simulink model "V2x_TC_TrafficLights_cs"  (Simulink 7.6)
settings: Solver=ode5  SolverMode=SingleTasking  StartTime=0.0  StopTime=38  FixedStep=1/100  RelTol=1e-3
PreLoadFcn:
  | check_NUTS_defined
  | 
  | assignin('base',['model_closed_' bdroot],false);addpath('../includes_V2x');
InitFcn:
  | PreScanExperimentInitFcn(bdroot)
StopFcn:
  | PreScanExperimentStopFcn(bdroot);
  | 
undefined symbols (not defined in the file or in its folder): acceleration, actorID, actorType, DiscIntNeverNeededParam, GPS_alt, GPS_lat, GPS_long, intmax, lightBarInUse, messageID, Newton, publicActorType, Raphson, rotZ, sigbuilder_block, sireneInUse, trafficLightPriority, turnAdvice, velocity, x_ini_VRML, y_ini_VRML, yawRate, z_ini_VRML
libraries: simulink x172, PreScanSimulinkLibrary x20, PreScanUsefulBlocks x11, simulink_extras x9, simulink_need_slupdate x1
(part 27 of 27)

== system V2x_TC_TrafficLights_cs/Audi_A8_1/Traffic Controller/Intersection warning controller/Enabled Subsystem/Intersection/Intersection collision warning controller/For Each Subsystem ==
  actorType : Inport  PortDimensions=1  Partition=on  PartitionDimension=1  PartitionWidth=1
  WM : Inport  Port=2  Partition=off
  Rcross : Inport  Port=3  Partition=off
  target range : Inport  Port=4  Partition=on  PartitionDimension=1  PartitionWidth=1
  target angle : Inport  Port=5  Partition=on  PartitionDimension=1  PartitionWidth=1
  target intention : Inport  Port=6  Partition=on  PartitionDimension=1  PartitionWidth=1
  target heading : Inport  Port=7  Partition=on  PartitionDimension=1  PartitionWidth=1
  targetVelocity : Inport  Port=8  PortDimensions=1  Partition=on  PartitionDimension=1  PartitionWidth=1
  For Each : ForEach  DisableCoverage=on  IndexMode=One-based
  If : If  NumInputs=2  IfExpression=(u1==1 | u1==2 | u1 ==3) & (u2~=0)
  If Action Subsystem : Subsystem  TreatAsAtomicUnit=on
  If Action Subsystem1 : Subsystem  TreatAsAtomicUnit=on
  Merge : Merge
  Merge1 : Merge
  Merge2 : Merge
  warning left lane : Outport  ConcatenationDimension=1
  warning straight lane : Outport  Port=2  ConcatenationDimension=1
  warning right lane : Outport  Port=3  ConcatenationDimension=1
  wires:
  actorType/1 -> If/1
  If/1 -> If Action Subsystem/Action
  If/2 -> If Action Subsystem1/Action
  WM/1 -> If Action Subsystem/1(WM)
  Rcross/1 -> If Action Subsystem/2(Rcross)
  target range/1 -> If Action Subsystem/3(target range)
  target angle/1 -> If Action Subsystem/4(target angle)
  target intention/1 -> If Action Subsystem/5(target intention)
  target heading/1 -> If Action Subsystem/6(target heading)
  If Action Subsystem/1(warning left lane) -> Merge/1
  If Action Subsystem/2(warning straight lane) -> Merge1/1
  If Action Subsystem/3(warning right lane) -> Merge2/1
  If Action Subsystem1/1(warning left lane) -> Merge/2
  If Action Subsystem1/2(warning straight lane) -> Merge1/2
  If Action Subsystem1/3(warning right lane) -> Merge2/2
  Merge/1 -> warning left lane/1
  Merge1/1 -> warning straight lane/1
  Merge2/1 -> warning right lane/1
  targetVelocity/1 -> If/2

== system V2x_TC_TrafficLights_cs/Audi_A8_1/Traffic Controller/Intersection warning controller/Enabled Subsystem/Intersection/Intersection collision warning controller/host intention translation ==
  host intention : Inport
  Bias : Bias  Bias=2
  Multiport Switch : MultiPortSwitch  zeroidx=off
  left_turning : Constant  Value=[0 1 1 1 1 1 0 1 1]
  right_turning : Constant  Value=[0 1 0 0 0 1 0 0 0]
  straight : Constant  Value=[0 1 1 0 0 1 1 1 1]
  WM : Outport
  wires:
  right_turning/1 -> Multiport Switch/4
  straight/1 -> Multiport Switch/3
  left_turning/1 -> Multiport Switch/2
  host intention/1 -> Bias/1
  Bias/1 -> Multiport Switch/1
  Multiport Switch/1 -> WM/1

== system V2x_TC_TrafficLights_cs/Audi_A8_1/Traffic Controller/Traffic signs and  traffic lights controller/Enabled Subsystem/major object selection lights/If Action Subsystem/While Iterator Subsystem ==
  cond : Inport
  Compare To Zero : simulink/Logic and Bit Operations/Compare To Zero  SystemSampleTime=-1  relop===  LogicOutDataTypeMode=uint8  ZeroCross=off
  Selector : Selector  NumberOfDimensions=2  InputPortWidth=3  IndexOptions=Index vector (port),Index vector (dialog)  Indices=[1 3],1  OutputSizes=1,1
  While Iterator : WhileIterator  MaxIters=-1  WhileBlockType=do-while  ShowIterationPort=on  OutputDataType=double
  idx : Outport
  wires:
  Selector/1 -> Compare To Zero/1
  cond/1 -> Selector/1
  While Iterator/1 -> idx/1, Selector/2
  Compare To Zero/1 -> While Iterator/1

== system V2x_TC_TrafficLights_cs/Audi_A8_1/Traffic Controller/Traffic signs and  traffic lights controller/Enabled Subsystem/major object selection signs/If Action Subsystem/Traffic signs warning ==
  MessageID : Inport
  hostVelocity : Inport  Port=2
  Compare To Zero : simulink/Logic and Bit Operations/Compare To Zero  SystemSampleTime=-1  relop=>  LogicOutDataTypeMode=uint8  ZeroCross=off
  Data Type Conversion : DataTypeConversion
  Gain : Gain  Gain=3.6
  Logical Operator : Logic  AllPortsSameDT=off  OutDataTypeStr=boolean
  Relational Operator : RelationalOperator  Operator=<  OutDataTypeStr=boolean
  message to speed limit conversion : Bias  Bias=-1000
  Warning : Outport
  wires:
  Logical Operator/1 -> Data Type Conversion/1
  Compare To Zero/1 -> Logical Operator/1
  Relational Operator/1 -> Logical Operator/2
  Gain/1 -> Relational Operator/2
  hostVelocity/1 -> Gain/1
  message to speed limit conversion/1 -> Compare To Zero/1, Relational Operator/1
  MessageID/1 -> message to speed limit conversion/1
  Data Type Conversion/1 -> Warning/1

== system V2x_TC_TrafficLights_cs/Audi_A8_1/Traffic Controller/Traffic signs and  traffic lights controller/Enabled Subsystem/major object selection signs/If Action Subsystem/While Iterator Subsystem ==
  cond : Inport
  Compare To Zero : simulink/Logic and Bit Operations/Compare To Zero  SystemSampleTime=-1  relop===  LogicOutDataTypeMode=uint8  ZeroCross=off
  Selector : Selector  NumberOfDimensions=2  InputPortWidth=3  IndexOptions=Index vector (port),Index vector (dialog)  Indices=[1 3],1  OutputSizes=1,1
  While Iterator : WhileIterator  MaxIters=-1  WhileBlockType=do-while  ShowIterationPort=on  OutputDataType=double
  idx : Outport
  wires:
  Selector/1 -> Compare To Zero/1
  cond/1 -> Selector/1
  While Iterator/1 -> idx/1, Selector/2
  Compare To Zero/1 -> While Iterator/1

== system V2x_TC_TrafficLights_cs/Audi_A8_1/Traffic Controller/Intersection warning controller/Enabled Subsystem/Intersection/Intersection collision warning controller/For Each Subsystem/If Action Subsystem ==
  WM : Inport
  Rcross : Inport  Port=2
  target range : Inport  Port=3
  target angle : Inport  Port=4
  target intention : Inport  Port=5
  target heading : Inport  Port=6
  Action Port : ActionPort
  Decision Algorithm : Stateflow chart  TreatAsAtomicUnit=on
  warning left lane : Outport  InitialOutput=false(1)
  warning straight lane : Outport  Port=2  InitialOutput=false(1)
  warning right lane : Outport  Port=3  InitialOutput=false(1)
  wires:
  Decision Algorithm/1(wLeft) -> warning left lane/1
  Rcross/1 -> Decision Algorithm/2(Rcross)
  target range/1 -> Decision Algorithm/3(range)
  Decision Algorithm/2(wStraight) -> warning straight lane/1
  target angle/1 -> Decision Algorithm/4(angle)
  target intention/1 -> Decision Algorithm/5(intention)
  Decision Algorithm/3(wRight) -> warning right lane/1
  target heading/1 -> Decision Algorithm/6(heading)
  WM/1 -> Decision Algorithm/1(WM)

== system V2x_TC_TrafficLights_cs/Audi_A8_1/Traffic Controller/Intersection warning controller/Enabled Subsystem/Intersection/Intersection collision warning controller/For Each Subsystem/If Action Subsystem1 ==
  Action Port : ActionPort
  Constant : Constant  Value=false(1)
  Constant1 : Constant  Value=false(1)
  Constant2 : Constant  Value=false(1)
  warning left lane : Outport  InitialOutput=false(1)
  warning straight lane : Outport  Port=2  InitialOutput=false(1)
  warning right lane : Outport  Port=3  InitialOutput=false(1)
  wires:
  Constant/1 -> warning left lane/1
  Constant1/1 -> warning straight lane/1
  Constant2/1 -> warning right lane/1

== stateflow chart Audi_A8_1/Traffic Controller/Intersection warning controller/Enabled Subsystem/Intersection/Intersection collision warning controller/For Each Subsystem/If Action Subsystem/Decision Algorithm ==
  state controller
    state Lane3
      state Straight
        en: wRight=WM[8];
      state RightTurn
        en: wRight=WM[7];
      state LeftTurn
        en: wRight=WM[9];
      (junction 19) -> controller.Lane3.LeftTurn [order 2] : [intention<0]
      (junction 19) -> controller.Lane3.Straight [order 3] : [intention==0]
      (default) -> (junction 19) [order 1]
      (default) -> controller.Lane3.Straight [order 2]
      (junction 19) -> controller.Lane3.RightTurn [order 1] : [intention>0]
    state Lane2
      state RightTurn
        en: wStraight=WM[4];
      state LeftTurn
        en: wStraight=WM[6];
      state Straight
        en: wStraight=WM[5];
      (junction 18) -> controller.Lane2.Straight [order 2] : [intention==0]
      (default) -> controller.Lane2.Straight [order 2]
      (junction 18) -> controller.Lane2.RightTurn [order 3] : [intention>0]
      (junction 18) -> controller.Lane2.LeftTurn [order 1] : [intention<0]
      (default) -> (junction 18) [order 1]
    state Lane1
      state Straight
        en: wLeft=WM[2];
      state LeftTurn
        en: wLeft=WM[3];
      state RightTurn
        en: wLeft=WM[1];
      (junction 17) -> controller.Lane1.RightTurn [order 3] : [intention>0]
      (junction 17) -> controller.Lane1.LeftTurn [order 1] : [intention<0]
      (junction 17) -> controller.Lane1.Straight [order 2] : [intention==0]
      (default) -> controller.Lane1.Straight [order 2]
      (default) -> (junction 17) [order 1]
    state Enter
    controller.Lane1.LeftTurn -> Enter
    controller.Lane3.RightTurn -> Enter
    (default) -> Enter : {wLeft=0;
        | wStraight=0;
        | wRight=0;}
    controller.Lane3.Straight -> Enter
    controller.Lane3.LeftTurn -> Enter
    Enter -> Enter [order 4] : {wLeft=0;
        | wStraight=0;
        | wRight=0;}
    controller.Lane1.RightTurn -> Enter
    Enter -> Lane3 [order 2] : [angle>-90 && angle<-45 && range<Rcross...
        | && (heading>45 && heading<135)]
    controller.Lane1.Straight -> Enter
    controller.Lane2.RightTurn -> Enter
    controller.Lane2.LeftTurn -> Enter
    Enter -> Lane2 [order 1] : [angle>0 && angle<45 && range<Rcross...
        | && heading>135 && heading<225]
    Enter -> Lane1 [order 3] : [angle>90 && angle<135 && range<Rcross...
        | && heading>225 && heading<315]
    controller.Lane2.Straight -> Enter
  data: WM input double size=9; Rcross input double; range input double; angle input double; intention input double; heading input double; wLeft output boolean size=1 =false(1); wStraight output boolean size=1 =false(1); wRight output boolean size=1 =false(1)

== matlab function Audi_A8_1/Traffic Controller/Intersection warning controller/Enabled Subsystem/sort using ID/Embedded MATLAB Function ==
  data: y output double; ids input double
function y = fcn(ids)
% Sorts ID vector

[~,y] = sort(ids,'descend');

end

== matlab function Audi_A8_1/Rx_Noise/Embedded MATLAB Function ==
  data: CAM_warning input double; CAM output double; CAM_messageID input double; CAM_vehicleID input double; CAM_vehicleType input double; CAM_publicVehicleType input double; CAM_lightBarInUse input double; CAM_sireneInUse input double; CAM_velocity input double; CAM_acceleration input double; CAM_yawRate input double; CAM_turnAdvice input double; CAM_trafficLightPriority input double; CAM_GPSlat input double; CAM_GPSlong input double; CAM_GPSalt input double; CAM_rotZ input double
function CAM = fcn(CAM_warning, CAM_messageID, CAM_vehicleID, CAM_vehicleType, CAM_publicVehicleType, CAM_lightBarInUse, CAM_sireneInUse, CAM_velocity, CAM_acceleration, CAM_yawRate, CAM_turnAdvice, CAM_trafficLightPriority, CAM_GPSlat, CAM_GPSlong, CAM_GPSalt, CAM_rotZ)
%#eml

CAM = [CAM_warning, CAM_messageID, CAM_vehicleID, CAM_vehicleType, CAM_publicVehicleType, CAM_lightBarInUse, CAM_sireneInUse, CAM_velocity, CAM_acceleration, CAM_yawRate, CAM_turnAdvice, CAM_trafficLightPriority, CAM_GPSlat, CAM_GPSlong, CAM_GPSalt, CAM_rotZ];

== matlab function Audi_A8_1/Rx_Noise/Embedded MATLAB Function1 ==
  data: CAM_warning output double; CAM input double; CAM_messageID output double; CAM_vehicleID output double; CAM_vehicleType output double; CAM_publicVehicleType output double; CAM_lightBarInUse output double; CAM_sireneInUse output double; CAM_velocity output double; CAM_acceleration output double; CAM_yawRate output double; CAM_turnAdvice output double; CAM_trafficLightPriority output double; CAM_GPSlat output double; CAM_GPSlong output double; CAM_GPSalt output double; CAM_rotZ output double
function [CAM_warning, CAM_messageID, CAM_vehicleID, CAM_vehicleType, CAM_publicVehicleType, CAM_lightBarInUse, CAM_sireneInUse, CAM_velocity, CAM_acceleration, CAM_yawRate, CAM_turnAdvice, CAM_trafficLightPriority, CAM_GPSlat, CAM_GPSlong, CAM_GPSalt, CAM_rotZ] = fcn(CAM)
%#eml

CAM_warning = CAM(:, 1);
CAM_messageID = CAM(:, 2); 
CAM_vehicleID = CAM(:, 3);
CAM_vehicleType = CAM(:, 4);
CAM_publicVehicleType = CAM(:, 5);
CAM_lightBarInUse = CAM(:, 6);
CAM_sireneInUse = CAM(:, 7);
CAM_velocity = CAM(:, 8);
CAM_acceleration = CAM(:, 9);
CAM_yawRate = CAM(:, 10);
CAM_turnAdvice = CAM(:, 11);
CAM_trafficLightPriority = CAM(:, 12);
CAM_GPSlat = CAM(:, 13);
CAM_GPSlong = CAM(:, 14);
CAM_GPSalt = CAM(:, 15);
CAM_rotZ = CAM(:, 16);
